simulink model "simuModel"  (R2017a)
settings: Solver=VariableStepAuto  StartTime=0.0  StopTime=30  RelTol=1e-3
libraries: dsparch4 x8, simulink x8, dspsnks4 x1, dspvision x1
settings Model: Creator=achar  LastModifiedBy=achar

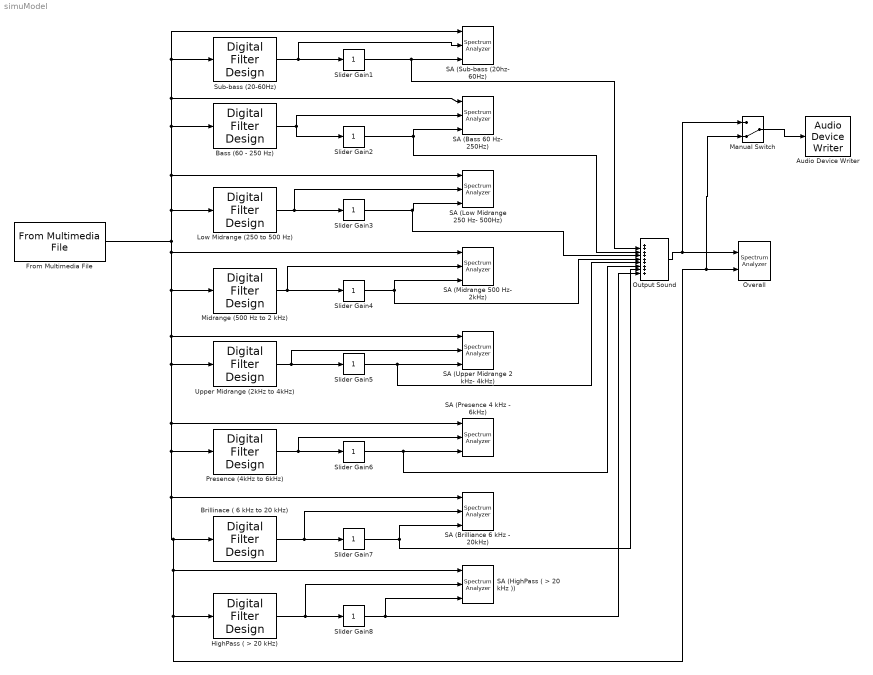
== system simuModel ==
  " Midrange (500 Hz to 2 kHz) " : dsparch4/Digital Filter Design  mwdsp_browser_bugfix_var=off
  Audio Device Writer : dspsnks4/Audio Device Writer  Driver=DirectSound  Device=Default  InheritSampleRate=on  SampleRate=44100  BitDepth=16-bit integer  BufferSize=4096  NumBuffers=10  DefaultOutputChannelMapping=on  OutputChannelMapping=1:2  OutputNumUnderrunSamples=off  DriverIdx=1  DeviceWarnFlag=''
  "Bass (60 - 250 Hz) " : dsparch4/Digital Filter Design  mwdsp_browser_bugfix_var=off
  "Brillinace ( 6 kHz to 20 kHz) " : dsparch4/Digital Filter Design  mwdsp_browser_bugfix_var=off
  From Multimedia File : dspvision/From Multimedia File  inputFilename=C:\Users\achar\OneDrive\Desktop\project\BE_OE.mp3  loop=on  numPlays=inf  outputStreams=Audio only  videoDataType=single  audioDataType=double  inheritSampleTime=on  userDefinedSampleTime=1/30  noAudioOutput=off  isIntensityVideo=off  fourcc=RGB  colorVideoFormat=Separate color signals  outputEOF=off  dataOrg=Column-major  audioFrameSize=1024  computeAudioFrameSize=off  outSamplingMode=Sample based
      settings: SourceProductBaseCode=VP
  HighPass ( > 20 kHz) : dsparch4/Digital Filter Design  mwdsp_browser_bugfix_var=off
  "Low Midrange (250 to 500 Hz) " : dsparch4/Digital Filter Design  mwdsp_browser_bugfix_var=off
  Manual Switch : ManualSwitch  CurrentSetting=0
  "Output Sound " : Sum  Inputs=+++++++++
  "Overall " : SpectrumAnalyzer  NumInputPorts=2
  "Presence (4kHz to 6kHz) " : dsparch4/Digital Filter Design  mwdsp_browser_bugfix_var=off
  "SA (Bass 60 Hz- 250Hz) " : SpectrumAnalyzer  NumInputPorts=3
  SA (Brilliance 6 kHz - 20kHz) : SpectrumAnalyzer  NumInputPorts=3
  "SA (HighPass ( > 20 kHz )) " : SpectrumAnalyzer  NumInputPorts=3
  SA (Low Midrange 250 Hz- 500Hz) : SpectrumAnalyzer  NumInputPorts=3
  SA (Midrange 500 Hz- 2kHz) : SpectrumAnalyzer  NumInputPorts=3
  SA (Presence 4 kHz - 6kHz) : SpectrumAnalyzer  NumInputPorts=3
  "SA (Sub-bass (20hz- 60Hz) " : SpectrumAnalyzer  NumInputPorts=3
  SA (Upper Midrange 2 kHz- 4kHz) : SpectrumAnalyzer  NumInputPorts=3
  Slider Gain{1..8} (8 alike) : simulink/Math Operations/Slider Gain  gain=1  low=-15  high=15
  "Sub-bass (20-60Hz) " : dsparch4/Digital Filter Design  mwdsp_browser_bugfix_var=off
  Upper Midrange (2kHz to 4kHz) : dsparch4/Digital Filter Design  mwdsp_browser_bugfix_var=off
  wires:
  "Sub-bass (20-60Hz) "/1 -> Slider Gain1/1, "SA (Sub-bass (20hz- 60Hz) "/2
  "Bass (60 - 250 Hz) "/1 -> Slider Gain2/1, "SA (Bass 60 Hz- 250Hz) "/2
  "Low Midrange (250 to 500 Hz) "/1 -> Slider Gain3/1, SA (Low Midrange 250 Hz- 500Hz)/2
  " Midrange (500 Hz to 2 kHz) "/1 -> Slider Gain4/1, SA (Midrange 500 Hz- 2kHz)/2
  Upper Midrange (2kHz to 4kHz)/1 -> Slider Gain5/1, SA (Upper Midrange 2 kHz- 4kHz)/2
  "Presence (4kHz to 6kHz) "/1 -> Slider Gain6/1, SA (Presence 4 kHz - 6kHz)/2
  Slider Gain1/1 -> "Output Sound "/2, "SA (Sub-bass (20hz- 60Hz) "/3
  Slider Gain2/1 -> "Output Sound "/3, "SA (Bass 60 Hz- 250Hz) "/3
  "Brillinace ( 6 kHz to 20 kHz) "/1 -> Slider Gain7/1, SA (Brilliance 6 kHz - 20kHz)/2
  "Output Sound "/1 -> "Overall "/1, Manual Switch/1
  From Multimedia File/1 -> "SA (Bass 60 Hz- 250Hz) "/1, "SA (Sub-bass (20hz- 60Hz) "/1, "Sub-bass (20-60Hz) "/1, "Bass (60 - 250 Hz) "/1, SA (Low Midrange 250 Hz- 500Hz)/1, "Low Midrange (250 to 500 Hz) "/1, SA (Midrange 500 Hz- 2kHz)/1, " Midrange (500 Hz to 2 kHz) "/1, SA (Upper Midrange 2 kHz- 4kHz)/1, Upper Midrange (2kHz to 4kHz)/1, SA (Presence 4 kHz - 6kHz)/1, SA (Brilliance 6 kHz - 20kHz)/1, "SA (HighPass ( > 20 kHz )) "/1, Manual Switch/2, "Overall "/2, HighPass ( > 20 kHz)/1, "Brillinace ( 6 kHz to 20 kHz) "/1, "Presence (4kHz to 6kHz) "/1
  Slider Gain4/1 -> "Output Sound "/5, SA (Midrange 500 Hz- 2kHz)/3
  Slider Gain5/1 -> "Output Sound "/6, SA (Upper Midrange 2 kHz- 4kHz)/3
  Slider Gain6/1 -> "Output Sound "/7, SA (Presence 4 kHz - 6kHz)/3
  Slider Gain7/1 -> "Output Sound "/8, SA (Brilliance 6 kHz - 20kHz)/3
  Slider Gain3/1 -> "Output Sound "/4, SA (Low Midrange 250 Hz- 500Hz)/3
  Manual Switch/1 -> Audio Device Writer/1
  HighPass ( > 20 kHz)/1 -> "SA (HighPass ( > 20 kHz )) "/2, Slider Gain8/1
  Slider Gain8/1 -> "SA (HighPass ( > 20 kHz )) "/3, "Output Sound "/9
